simulink model "TosAeb"  (R2021a) (continued)
== system TosAeb/FcwAebJa_Step_sys/ALG_AEBSystem/ALG_AEBController/AEB_JaObjCalcApp/JAVehTgtInfoCalc/AEBJAVehObjCalc/ObjPreSelectCondCalc ==
  AEBJaVehObj : Inport  OutDataTypeStr=Bus: BusAEBJAObjBus
  subjVel : Inport  Port=2
  Bus Selector : BusSelector  OutputSignals=ObjectID,ObjectExistProb,ObjectMovingState,ObjectMovingOrientation,ObjectTypeCameraConfirmed,ObjectType,dxVariance,vx,dy,dx
  If : If  IfExpression=u1
  Merge : Merge
  ObjIsInvalidCalc : Subsystem
  TgtPreSelect : Subsystem
  TgtPreSelectCondDefault : Subsystem  TreatAsAtomicUnit=on
  ObjPreSelectCondFufilled : Outport  OutDataTypeStr=boolean  LockScale=on
  wires:
  AEBJaVehObj/1 -> Bus Selector/1
  Bus Selector/2 -> TgtPreSelect/1(ObjectExistProb)  "<ObjectExistProb>"
  Bus Selector/3 -> TgtPreSelect/2(ObjectMovingState)  "<ObjectMovingState>"
  Bus Selector/4 -> TgtPreSelect/3(ObjectMovingOrientation)  "<ObjectMovingOrientation>"
  Bus Selector/5 -> TgtPreSelect/4(ObjectTypeCameraConfirmed)  "<ObjectTypeCameraConfirmed>"
  Bus Selector/6 -> TgtPreSelect/5(ObjectType)  "<ObjectType>"
  Bus Selector/7 -> TgtPreSelect/6(dxVariance)  "<dxVariance>"
  ObjIsInvalidCalc/1(ObjIsInvalid) -> If/1
  If/1 -> TgtPreSelectCondDefault/Action
  Bus Selector/1 -> ObjIsInvalidCalc/1(ObjectID)  "<ObjectID>"
  If/2 -> TgtPreSelect/Action
  TgtPreSelect/1(ObjPreSelectCondFufilled) -> Merge/2
  Merge/1 -> ObjPreSelectCondFufilled/1
  TgtPreSelectCondDefault/1(ObjPreSelectCondFufilled) -> Merge/1
  Bus Selector/8 -> TgtPreSelect/7(vx)  "<vx>"
  Bus Selector/9 -> TgtPreSelect/8(dy)  "<dy>"
  Bus Selector/10 -> TgtPreSelect/9(dx)  "<dx>"
  subjVel/1 -> TgtPreSelect/10(subjVel)  "subjVel"

== system TosAeb/FcwAebJa_Step_sys/ALG_AEBSystem/ALG_AEBController/AEB_JaObjCalcApp/JAVehTgtInfoCalc/JAVehCollisonAssessInfoCalc/JaVehCollisonAssessInfoCalc ==
  vx : Inport  OutDataTypeStr=single
  vy : Inport  Port=2  OutDataTypeStr=single
  dx : Inport  Port=3  OutDataTypeStr=single
  dy : Inport  Port=4  OutDataTypeStr=single
  dxFrontBumper : Inport  Port=5  OutDataTypeStr=single
  tPredictTime : Inport  Port=6  OutDataTypeStr=single
  subjYawRate : Inport  Port=7  OutDataTypeStr=single
  subjVel : Inport  Port=8  OutDataTypeStr=single
  subjLongAccel : Inport  Port=9  OutDataTypeStr=single
  Action Port : ActionPort  ActionPortLabel=else
  Bus Creator : BusCreator  Inputs=3
  VehDistBrakeFullCalc : Subsystem
  VelAbsTgtCorrCalc : Subsystem
  distRadical2CollsionCalc : Subsystem
  tBrakeingCalc : Subsystem  Variant=on
  JAVehCollisonAssessInfo : Outport
  wires:
  Bus Creator/1 -> JAVehCollisonAssessInfo/1
  subjYawRate/1 -> VelAbsTgtCorrCalc/5(subjYawRate)
  subjVel/1 -> VelAbsTgtCorrCalc/6(subjVel), tBrakeingCalc/2(subjVel), VehDistBrakeFullCalc/2(subjVel)
  tPredictTime/1 -> distRadical2CollsionCalc/4(tPredictTime)
  dy/1 -> VelAbsTgtCorrCalc/3(dy)
  dxFrontBumper/1 -> VelAbsTgtCorrCalc/4(dxFrontBumper)
  vx/1 -> VelAbsTgtCorrCalc/1(vx)
  vy/1 -> VelAbsTgtCorrCalc/2(vy)
  dx/1 -> distRadical2CollsionCalc/3(dx)
  VehDistBrakeFullCalc/2(tBrake) -> tBrakeingCalc/1(tBarke)
  distRadical2CollsionCalc/2(restLatDist2Collsion) -> Bus Creator/2  "restLatDist2Collsion"
  tBrakeingCalc/1(TTB) -> Bus Creator/3  "TTB"
  VelAbsTgtCorrCalc/2(vyAbsTgtCorr) -> distRadical2CollsionCalc/2(vyAbsTgtCorr)
  VehDistBrakeFullCalc/1(distBrake) -> distRadical2CollsionCalc/5(distBrake)  "longDistBraking"
  distRadical2CollsionCalc/1(initialLatDist) -> Bus Creator/1  "initialLatDist"
  subjLongAccel/1 -> VehDistBrakeFullCalc/1(subjLongAccel)
  VelAbsTgtCorrCalc/1(vxAbsTgtCorr) -> distRadical2CollsionCalc/1(vxAbsTgtCorr)

== system TosAeb/FcwAebJa_Step_sys/ALG_AEBSystem/ALG_AEBController/AEB_JaObjCalcApp/JAVehTgtInfoCalc/JAVehCollisonAssessInfoCalc/JaVehCollisonAssessInfoDefault ==
  Action Port : ActionPort  ActionPortLabel=if(u1)
  Bus Creator : BusCreator  Inputs=3
  Constant1 : Constant  Value=255  OutDataTypeStr=single
  Constant2 : Constant  Value=255  OutDataTypeStr=single
  Constant3 : Constant  Value=255  OutDataTypeStr=single
  JAVehCollisonAssessInfo : Outport
  wires:
  Constant1/1 -> Bus Creator/1  "initialLatDist"
  Constant2/1 -> Bus Creator/2  "restLatDist2Collsion"
  Bus Creator/1 -> JAVehCollisonAssessInfo/1
  Constant3/1 -> Bus Creator/3  "TTB"

== system TosAeb/FcwAebJa_Step_sys/ALG_AEBSystem/ALG_AEBController/AEB_JaObjCalcApp/JAVehTgtInfoCalc/JAVehCollisonAssessInfoCalc/ObjIsInvalidJudge ==
  ObjectID : Inport
  Compare To Constant : simulink/Logic and Bit Operations/Compare To Constant  relop===  const=CNobjInvalidID  OutDataTypeStr=boolean
  ObjIsInvalid : Outport
  wires:
  ObjectID/1 -> Compare To Constant/1
  Compare To Constant/1 -> ObjIsInvalid/1

== system TosAeb/FcwAebJa_Step_sys/ALG_AEBSystem/ALG_AEBController/AEB_JaObjCalcApp/JAVehTgtInfoCalc/TargetFinalSelect/tgtSelect ==
  JAVehObjCalcInfo : Inport
  ObjPreSelectCondFufilled : Inport  Port=2
  subjVel : Inport  Port=3
  SteerWheelAngle_Deg : Inport  Port=4
  JAVehPrefillModSts : Inport  Port=5
  JAVehAEBfullModSts : Inport  Port=6
  Bus Selector : BusSelector  OutputSignals=AEBJAVehObj.ObjectID
  Constant : Constant  Value=P_AEB_JaVehCA_SelectSubjVelThres
  Constant1 : Constant  Value=P_AEB_JaVehCA_SelectStrWheelAngleAbsThres
  Unit Delay : UnitDelay  InitialCondition=1  SampleTime=-1  HasFrameUpgradeWarning=on
  Unit Delay1 : UnitDelay  InitialCondition=1  SampleTime=-1  HasFrameUpgradeWarning=on
  tgtObjFlow : Stateflow chart  TreatAsAtomicUnit=on
  JAVehTgtObjInfo : Outport
  wires:
  tgtObjFlow/1(JAVehTgtObjInfo) -> Bus Selector/1, JAVehTgtObjInfo/1  "tgtObj"
  JAVehObjCalcInfo/1 -> tgtObjFlow/1(JAVehObjCalcInfo)
  ObjPreSelectCondFufilled/1 -> tgtObjFlow/2(ObjPreSelectCondFufilled)
  subjVel/1 -> tgtObjFlow/3(subjVel)
  SteerWheelAngle_Deg/1 -> tgtObjFlow/4(SteerWheelAngle_Deg)
  tgtObjFlow/2(tgtIndex) -> Unit Delay/1
  Unit Delay/1 -> tgtObjFlow/10(tgtIndexK1)
  JAVehPrefillModSts/1 -> tgtObjFlow/5(JAVehAEBfullModSts)
  JAVehAEBfullModSts/1 -> tgtObjFlow/6(JAVehPrefillModSts)
  Bus Selector/1 -> Unit Delay1/1  "<ObjectID>"
  Unit Delay1/1 -> tgtObjFlow/9(TargetIDK1)
  Constant/1 -> tgtObjFlow/7(P_JaVehCA_SelectSubjVelThres)
  Constant1/1 -> tgtObjFlow/8(P_JaVehCA_SelectStrWheelAngleAbsThres)

== system TosAeb/FcwAebJa_Step_sys/ALG_AEBSystem/ALG_AEBController/AEB_JaObjCalcApp/JaCycTgtInfoCalc/JaCycCollisonAssessInfo/JaCycCollisonAssessInfoCalc ==
  vx : Inport  OutDataTypeStr=single
  vy : Inport  Port=2  OutDataTypeStr=single
  dx : Inport  Port=3  OutDataTypeStr=single
  dy : Inport  Port=4  OutDataTypeStr=single
  dxFrontBumper : Inport  Port=5  OutDataTypeStr=single
  tPredictTime : Inport  Port=6  OutDataTypeStr=single
  subjYawRate : Inport  Port=7  OutDataTypeStr=single
  subjVel : Inport  Port=8  OutDataTypeStr=single
  subjLongAccel : Inport  Port=9  OutDataTypeStr=single
  Action Port : ActionPort  ActionPortLabel=else
  Bus Creator : BusCreator  Inputs=4
  PedDistBrakeFullCalc : Subsystem
  Subsystem1 : Subsystem
  VelAbsTgtCorrCalc : Subsystem
  distRadical2CollsionCalc : Subsystem
  JaCycCollisonAssessInfo : Outport
  wires:
  Bus Creator/1 -> JaCycCollisonAssessInfo/1
  VelAbsTgtCorrCalc/2(vyAbsTgtCorr) -> distRadical2CollsionCalc/2(vyAbsTgtCorr), Subsystem1/2(vyAbsTargetCorr)
  tPredictTime/1 -> distRadical2CollsionCalc/5(tPredictTime)
  PedDistBrakeFullCalc/2(tBrake) -> Bus Creator/4  "TTB"
  subjLongAccel/1 -> PedDistBrakeFullCalc/1(subjLongAccel)
  dxFrontBumper/1 -> VelAbsTgtCorrCalc/4(dxFrontBumper)
  Subsystem1/1(TgtYawAngle) -> Bus Creator/1  "TgtYawAngle"
  subjYawRate/1 -> VelAbsTgtCorrCalc/5(subjYawRate)
  PedDistBrakeFullCalc/1(distBrake) -> Bus Creator/3  "distBraking"
  [dy] -> distRadical2CollsionCalc/4(dy)
  vy/1 -> VelAbsTgtCorrCalc/2(vy)
  dx/1 -> distRadical2CollsionCalc/3(dx)
  dy/1 -> [dy], VelAbsTgtCorrCalc/3(dy)
  distRadical2CollsionCalc/1(distRadical2Collsion) -> Bus Creator/2  "distRadical2Collsion"
  subjVel/1 -> VelAbsTgtCorrCalc/6(subjVel), PedDistBrakeFullCalc/2(subjVel)
  VelAbsTgtCorrCalc/1(vxAbsTgtCorr) -> distRadical2CollsionCalc/1(vxAbsTgtCorr), Subsystem1/1(vxAbsTargetCorr)
  vx/1 -> VelAbsTgtCorrCalc/1(vx)

== system TosAeb/FcwAebJa_Step_sys/ALG_AEBSystem/ALG_AEBController/AEB_JaObjCalcApp/JaCycTgtInfoCalc/JaCycCollisonAssessInfo/JaCycCollisonAssessInfoDefault ==
  Action Port : ActionPort  ActionPortLabel=if(u1)
  Bus Creator : BusCreator  Inputs=4
  Constant : Constant  Value=0  OutDataTypeStr=single
  Constant1 : Constant  Value=255  OutDataTypeStr=single
  Constant2 : Constant  Value=255  OutDataTypeStr=single
  Constant3 : Constant  Value=256  OutDataTypeStr=single
  JaCycCollisonAssessInfo : Outport
  wires:
  Constant2/1 -> Bus Creator/3  "distBraking"
  Constant1/1 -> Bus Creator/2  "distRadical2Collsion"
  Bus Creator/1 -> JaCycCollisonAssessInfo/1
  Constant/1 -> Bus Creator/1  "TgtYawAngle"
  Constant3/1 -> Bus Creator/4  "TTB"

== system TosAeb/FcwAebJa_Step_sys/ALG_AEBSystem/ALG_AEBController/AEB_JaObjCalcApp/JaCycTgtInfoCalc/JaCycCollisonAssessInfo/ObjIsInvalidJudge ==
  ObjectID : Inport
  Compare To Constant : simulink/Logic and Bit Operations/Compare To Constant  relop===  const=CNobjInvalidID  OutDataTypeStr=boolean
  ObjIsInvalid : Outport
  wires:
  ObjectID/1 -> Compare To Constant/1
  Compare To Constant/1 -> ObjIsInvalid/1

== system TosAeb/FcwAebJa_Step_sys/ALG_AEBSystem/ALG_AEBController/AEB_JaObjCalcApp/JaCycTgtInfoCalc/JaCycObjCalcInfoCalc/JaCycObjInfoCalc ==
  JaCycObj : Inport
  subjInfo : Inport  Port=2
  Action Port : ActionPort  ActionPortLabel=else
  Bus Creator : BusCreator  Inputs=3  OutDataTypeStr=Bus: BusAEBJaCycObjCalcInfo  NonVirtualBus=on
      settings: InheritFromInputs=off
  Bus Creator2 : BusCreator  Inputs=6  OutDataTypeStr=Bus: BusAEBJaCycObjAbsInfo  NonVirtualBus=on
      settings: InheritFromInputs=off
  Bus Selector2 : BusSelector  OutputSignals=dx,dy,vx,vy,ax,ObjectID,ObjectTypeCameraConfirmed,FR_ObjectID,FR_Dx,FR_Vy,FC_ObjectID,FC_Dx,FC_Vy
  Bus Selector3 : BusSelector  OutputSignals=subjLongAccel,subjYawRate,subjVel
  ObjCrossFlgCal : Subsystem
  SensorTgtInfo2AbsTgtInfoTrans1 : Subsystem
  "JaCycObjCalcInfo " : Outport  OutDataTypeStr=Bus: BusAEBJaCycObjCalcInfo
  wires:
  ObjCrossFlgCal/1(ObjColliInfo) -> Bus Creator/3  "BusAEBJAObjColliInfo"
  [ObjAbsInfo] -> Bus Creator/2  "BusAEBJAObjAbsInfo"
  Bus Creator2/1 -> [ObjAbsInfo], ObjCrossFlgCal/2(ObjAbsInfo)
  subjInfo/1 -> ObjCrossFlgCal/3(subjInfo), Bus Selector3/1
  JaCycObj/1 -> Bus Selector2/1, Bus Creator/1, ObjCrossFlgCal/1(JaCycObj)  "AEBJACycObj"
  Bus Creator/1 -> "JaCycObjCalcInfo "/1
  SensorTgtInfo2AbsTgtInfoTrans1/5(ObjFusQuality) -> Bus Creator2/5  "ObjFusQuality"
  Bus Selector2/8 -> SensorTgtInfo2AbsTgtInfoTrans1/8(FR_ObjectID)  "<FR_ObjectID>"
  SensorTgtInfo2AbsTgtInfoTrans1/6(ObjectYawAngleCorr) -> Bus Creator2/6  "ObjectYawAngleCorr"
  Bus Selector2/3 -> SensorTgtInfo2AbsTgtInfoTrans1/3(vx)  "<vx>"
  Bus Selector2/2 -> SensorTgtInfo2AbsTgtInfoTrans1/2(dy)  "<dy>"
  Bus Selector2/13 -> SensorTgtInfo2AbsTgtInfoTrans1/13(FC_Vy)  "<FC_Vy>"
  Bus Selector3/2 -> SensorTgtInfo2AbsTgtInfoTrans1/15(subjYawRate)  "<subjYawRate>"
  Bus Selector2/5 -> SensorTgtInfo2AbsTgtInfoTrans1/5(ax)  "<ax>"
  Bus Selector2/11 -> SensorTgtInfo2AbsTgtInfoTrans1/11(FC_ObjectID)  "<FC_ObjectID>"
  Bus Selector3/3 -> SensorTgtInfo2AbsTgtInfoTrans1/16(subjVel)  "<subjVel>"
  SensorTgtInfo2AbsTgtInfoTrans1/2(vxAbsObj) -> Bus Creator2/2  "vxAbsObj"
  Bus Selector2/6 -> SensorTgtInfo2AbsTgtInfoTrans1/6(ObjectID)  "<ObjectID>"
  Bus Selector2/1 -> SensorTgtInfo2AbsTgtInfoTrans1/1(dx)  "<dx>"
  Bus Selector3/1 -> SensorTgtInfo2AbsTgtInfoTrans1/14(subjLongAccel)  "<subjLongAccel>"
  Bus Selector2/9 -> SensorTgtInfo2AbsTgtInfoTrans1/9(FR_Dx)  "<FR_Dx>"
  SensorTgtInfo2AbsTgtInfoTrans1/4(vyRelCorr) -> Bus Creator2/4  "vyRelCorr"
  SensorTgtInfo2AbsTgtInfoTrans1/1(dxFrontBumper) -> Bus Creator2/1  "dxFrontBumper"
  Bus Selector2/7 -> SensorTgtInfo2AbsTgtInfoTrans1/7(ObjectTypeCameraConfirmed)  "<ObjectTypeCameraConfirmed>"
  SensorTgtInfo2AbsTgtInfoTrans1/3(axAbsObj) -> Bus Creator2/3  "axAbsObj"
  Bus Selector2/12 -> SensorTgtInfo2AbsTgtInfoTrans1/12(FC_Dx)  "<FC_Dx>"
  Bus Selector2/10 -> SensorTgtInfo2AbsTgtInfoTrans1/10(FR_Vy)  "<FR_Vy>"
  Bus Selector2/4 -> SensorTgtInfo2AbsTgtInfoTrans1/4(vy)  "<vy>"

== system TosAeb/FcwAebJa_Step_sys/ALG_AEBSystem/ALG_AEBController/AEB_JaObjCalcApp/JaCycTgtInfoCalc/JaCycObjCalcInfoCalc/JaCycObjInfoDefault ==
  Action Port : ActionPort  ActionPortLabel=if(u1)
  Constant : Constant  Value=JACycObjCalcInfoDefaultParams  OutDataTypeStr=Bus: BusAEBJaCycObjCalcInfo
  JaCycObjCalcInfo : Outport  OutDataTypeStr=Bus: BusAEBJaCycObjCalcInfo
  wires:
  Constant/1 -> JaCycObjCalcInfo/1

== system TosAeb/FcwAebJa_Step_sys/ALG_AEBSystem/ALG_AEBController/AEB_JaObjCalcApp/JaCycTgtInfoCalc/JaCycObjCalcInfoCalc/ObjPreSelectCondCalc ==
  JaObj : Inport  OutDataTypeStr=Bus: BusAEBJAObjBus
  Bus Selector : BusSelector  OutputSignals=ObjectID,ObjectExistProb,ObjectYawAngle,ObjectTypeCameraConfirmed,ObjectType,dxVariance,dx,dy
  If : If  IfExpression=u1
  Merge : Merge
  ObjIsInvalidCalc : Subsystem
  TgtPreSelectCondCalc : Subsystem
  TgtPreSelectCondCalc1 : Subsystem  TreatAsAtomicUnit=on
  ObjPreSelectCond : Outport  OutDataTypeStr=boolean  LockScale=on
  wires:
  JaObj/1 -> Bus Selector/1
  Bus Selector/1 -> ObjIsInvalidCalc/1(ObjectID)  "<ObjectID>"
  Bus Selector/2 -> TgtPreSelectCondCalc/1(ObjectExistProb)  "<ObjectExistProb>"
  Bus Selector/3 -> TgtPreSelectCondCalc/2(ObjectYawAngle)  "<ObjectYawAngle>"
  Bus Selector/4 -> TgtPreSelectCondCalc/3(ObjectTypeCameraComfirmed)  "<ObjectTypeCameraConfirmed>"
  Bus Selector/5 -> TgtPreSelectCondCalc/4(ObjectType)  "<ObjectType>"
  Bus Selector/6 -> TgtPreSelectCondCalc/5(dxVariance)  "<dxVariance>"
  Bus Selector/7 -> TgtPreSelectCondCalc/6(dx)  "<dx>"
  Bus Selector/8 -> TgtPreSelectCondCalc/7(dy)  "<dy>"
  TgtPreSelectCondCalc1/1(ObjPreSelectCondFufilled) -> Merge/1
  TgtPreSelectCondCalc/1(ObjPreSelectCondFufilled) -> Merge/2
  If/2 -> TgtPreSelectCondCalc/Action
  If/1 -> TgtPreSelectCondCalc1/Action
  ObjIsInvalidCalc/1(ObjIsInvalid) -> If/1
  Merge/1 -> ObjPreSelectCond/1

== system TosAeb/FcwAebJa_Step_sys/ALG_AEBSystem/ALG_AEBController/AEB_JaObjCalcApp/JaCycTgtInfoCalc/TargetSelectCalc/tgtSelectFlow ==
  JaCycObjCalcInfo : Inport
  ObjPreSelectCondFufilled : Inport  Port=2
  subjVel : Inport  Port=3
  SteerWheelAngle_Deg : Inport  Port=4
  JaCycPrefillModSts : Inport  Port=5
  JaCycAEBfullModSts : Inport  Port=6
  Bus Selector : BusSelector  OutputSignals=JaCycObj.ObjectID
  Constant : Constant  Value=P_AEB_JaCycCA_SelectStrWheelAngleAbsThres
  Constant1 : Constant  Value=P_AEB_JaCycCA_SelectSubjVelThres
  Unit Delay : UnitDelay  InitialCondition=1  SampleTime=-1  HasFrameUpgradeWarning=on
  Unit Delay1 : UnitDelay  SampleTime=-1  HasFrameUpgradeWarning=on
  tgtObjFlow : Stateflow chart  TreatAsAtomicUnit=on
  JaCycTgtObjInfo : Outport
  wires:
  JaCycObjCalcInfo/1 -> tgtObjFlow/1(JaCycObjCalcInfo)
  SteerWheelAngle_Deg/1 -> tgtObjFlow/4(SteerWheelAngle_Deg)
  Unit Delay1/1 -> tgtObjFlow/7(TargetIDK1)
  Bus Selector/1 -> Unit Delay1/1  "<ObjectID>"
  JaCycPrefillModSts/1 -> tgtObjFlow/5(JACycAEBfullModSts)
  tgtObjFlow/2(tgtIndex) -> Unit Delay/1
  Unit Delay/1 -> tgtObjFlow/8(tgtIndexK1)
  ObjPreSelectCondFufilled/1 -> tgtObjFlow/2(ObjPreSelectCondFufilled)
  subjVel/1 -> tgtObjFlow/3(subjVel)
  JaCycAEBfullModSts/1 -> tgtObjFlow/6(JACycPrefillModSts)
  tgtObjFlow/1(JaCycTgtObjInfo) -> Bus Selector/1, JaCycTgtObjInfo/1  "JaCycTgtObjInfo"
  Constant/1 -> tgtObjFlow/9(P_JaCycCA_SelectStrWheelAngleAbsThres)
  Constant1/1 -> tgtObjFlow/10(P_JaCycCA_SelectSubjVelThres)

== system TosAeb/FcwAebJa_Step_sys/ALG_AEBSystem/ALG_AEBController/AEB_LatObjInfoCalcApp/LatCycObjCalc/LatCycCollisonAssessInfoCalc/LatCycCollisonAssessInfoCalc ==
  ObjectID : Inport
  cycColliisonRiskProb : Inport  Port=2
  subLongAccel : Inport  Port=3
  subjVel : Inport  Port=4
  Action Port : ActionPort  ActionPortLabel=else
  Bus Creator : BusCreator  Inputs=4
  DistBrkCondCalc : Subsystem
  LatCollsionSeverityJudge : Subsystem
  latCollisonAssessInfo : Outport
  wires:
  ObjectID/1 -> LatCollsionSeverityJudge/1(ObjectID)
  cycColliisonRiskProb/1 -> LatCollsionSeverityJudge/2(cycColliisonRiskProb)
  subLongAccel/1 -> DistBrkCondCalc/1(subLongAccel)
  subjVel/1 -> DistBrkCondCalc/2(subjVel)
  LatCollsionSeverityJudge/1(latCollsionSeverity) -> Bus Creator/1  "latCollisionSeverity"
  Bus Creator/1 -> latCollisonAssessInfo/1  "latCollisonAssessInfo"
  DistBrkCondCalc/2(distBraking4FullBrake) -> Bus Creator/3  "distBraking4FullBrake"
  DistBrkCondCalc/3(distSecure) -> Bus Creator/4  "distSecure"
  DistBrkCondCalc/1(distBraking4PartBrake) -> Bus Creator/2  "distBraking4PartBrake"

== system TosAeb/FcwAebJa_Step_sys/ALG_AEBSystem/ALG_AEBController/AEB_LatObjInfoCalcApp/LatCycObjCalc/LatCycCollisonAssessInfoCalc/LatCycCollisonAssessInfoDefault ==
  Action Port : ActionPort  ActionPortLabel=if(u1)
  Bus Creator : BusCreator  Inputs=4
  Constant : Constant  Value=CollisionSeverity.None
  Constant1 : Constant  Value=CNNull
  Constant2 : Constant  Value=CNNull
  Constant3 : Constant  Value=CNNull
  latCollisonAssessInfo : Outport
  wires:
  Constant/1 -> Bus Creator/1  "latCollisionSeverity"
  Bus Creator/1 -> latCollisonAssessInfo/1  "latCollisonAssessInfo"
  Constant2/1 -> Bus Creator/4  "distSecure"
  Constant1/1 -> Bus Creator/2  "distBraking4PartBrake"
  Constant3/1 -> Bus Creator/3  "distBraking4FullBrake"

== system TosAeb/FcwAebJa_Step_sys/ALG_AEBSystem/ALG_AEBController/AEB_LatObjInfoCalcApp/LatCycObjCalc/LatCycCollisonAssessInfoCalc/ObjIsInvalidJudge ==
  ObjectID : Inport
  Compare To Constant : simulink/Logic and Bit Operations/Compare To Constant  relop===  const=CNobjInvalidID  OutDataTypeStr=boolean
  ObjIsInvalid : Outport
  wires:
  ObjectID/1 -> Compare To Constant/1
  Compare To Constant/1 -> ObjIsInvalid/1

== system TosAeb/FcwAebJa_Step_sys/ALG_AEBSystem/ALG_AEBController/AEB_LatObjInfoCalcApp/LatCycObjCalc/LatCycObjCalc/TgtPreSelectCalc ==
  latCycObjInfo : Inport  OutDataTypeStr=Bus: BusAEBLatObj  PortDimensions=1  BusOutputAsStruct=on
  subjVel : Inport  Port=2
  Bus Selector : BusSelector  OutputSignals=ObjectID,dx,dy,ObjectExistProb,dyVariance,ObjectMovingOrientation,ObjectType,ObjectTypeCameraConfirmed,vx
  If : If  IfExpression=u1
  Merge : Merge
  ObjIsInvalidJudge : Subsystem
  TgtPreSelect : Subsystem
  TgtPreSelectDefault : Subsystem  TreatAsAtomicUnit=on
  ObjPreSelectCond : Outport
  wires:
  If/2 -> TgtPreSelect/Action
  Merge/1 -> ObjPreSelectCond/1
  TgtPreSelect/1(ObjPreSelectCond) -> Merge/2
  TgtPreSelectDefault/1(ObjPreSelectCond) -> Merge/1
  If/1 -> TgtPreSelectDefault/Action
  Bus Selector/1 -> ObjIsInvalidJudge/1(ObjectID)  "<ObjectID>"
  ObjIsInvalidJudge/1(ObjIsInvalid) -> If/1
  latCycObjInfo/1 -> Bus Selector/1
  Bus Selector/2 -> TgtPreSelect/1(dx)  "<dx>"
  Bus Selector/3 -> TgtPreSelect/2(dy)  "<dy>"
  Bus Selector/4 -> TgtPreSelect/3(ObjectExistProb)  "<ObjectExistProb>"
  Bus Selector/5 -> TgtPreSelect/4(dyVariance)  "<dyVariance>"
  Bus Selector/6 -> TgtPreSelect/5(ObjectMovingOrientation)  "<ObjectMovingOrientation>"
  Bus Selector/7 -> TgtPreSelect/6(ObjectType)  "<ObjectType>"
  Bus Selector/8 -> TgtPreSelect/7(ObjectTypeCameraConfirmed)  "<ObjectTypeCameraConfirmed>"
  Bus Selector/9 -> TgtPreSelect/8(vx)  "<vx>"
  subjVel/1 -> TgtPreSelect/9(subjVel)

== system TosAeb/FcwAebJa_Step_sys/ALG_AEBSystem/ALG_AEBController/AEB_LatObjInfoCalcApp/LatCycObjCalc/LatCycObjCalc/latCycObjInfoCalc ==
  subjInfo : Inport
  latCycObjInfo : Inport  Port=2  OutDataTypeStr=Bus: BusAEBLatObj
  Action Port : ActionPort  ActionPortLabel=else
  Bus Creator : BusCreator  Inputs=6  OutDataTypeStr=Bus: BusLatCycObjCalcInfo  NonVirtualBus=on
      settings: InheritFromInputs=off
  Bus Creator1 : BusCreator  Inputs=5
  Bus Selector : BusSelector  OutputSignals=dx,vx,ax,vy,ObjectID,ObjectTypeCameraConfirmed,dy,FR_ObjectID,FR_Dx,FR_Dy,FR_Vy,FC_ObjectID,FC_Dx,FC_Dy,FC_Vy
  Bus Selector1 : BusSelector  OutputSignals=subjVel,subjLongAccel,subjYawRate
  Constant : Constant  Value=TRUE
  Constant1 : Constant  Value=P_AEB_LatCycCA_PreCollisionConfig
  ObjColiisionRiskProbCalc : Subsystem
  ObjCrossFlgCal : Subsystem
  SensorTgtInfo2AbsTgtInfoTrans : Subsystem
  Switch : Switch  Criteria=u2 ~= 0
  Terminator : Terminator
  Terminator1 : Terminator
  latCycCalcInfo : Outport  OutDataTypeStr=Bus: BusLatCycObjCalcInfo
  wires:
  [ObjAbsInfo] -> ObjCrossFlgCal/3(ObjAbsInfo)
  ObjColiisionRiskProbCalc/3(dyCollisionRiskProbOut) -> Terminator1/1
  ObjColiisionRiskProbCalc/2(dxCollisionRiskProbOut) -> Terminator/1
  subjInfo/1 -> ObjColiisionRiskProbCalc/3(subjInfo), ObjCrossFlgCal/1(subjInfo), Bus Selector1/1
  Bus Creator1/1 -> ObjColiisionRiskProbCalc/2(ObjAbsInfo), [ObjAbsInfo]
  Bus Creator/1 -> latCycCalcInfo/1
  ObjColiisionRiskProbCalc/1(cycCollisionRiskProb) -> Bus Creator/3  "cycCollisionRiskProb"
  [ObjAbsInfo] -> Bus Creator/2  "cycObjAbsInfo"
  Switch/1 -> Bus Creator/5  "cycPreCollisionFlg"
  latCycObjInfo/1 -> ObjCrossFlgCal/2(ObjInfoRaw), Bus Selector/1, ObjColiisionRiskProbCalc/1(ObjInfoRaw), Bus Creator/1  "latCycObjInfo"
  Bus Selector/11 -> SensorTgtInfo2AbsTgtInfoTrans/11(FR_Vy)  "<FR_Vy>"
  Bus Selector/6 -> SensorTgtInfo2AbsTgtInfoTrans/6(ObjectTypeCameraConfirmed)  "<ObjectTypeCameraConfirmed>"
  Bus Selector/8 -> SensorTgtInfo2AbsTgtInfoTrans/8(FR_ObjectID)  "<FR_ObjectID>"
  Bus Selector/5 -> SensorTgtInfo2AbsTgtInfoTrans/5(ObjectID)  "<ObjectID>"
  SensorTgtInfo2AbsTgtInfoTrans/4(vyCorr) -> Bus Creator1/4  "vyCorr"
  Bus Selector1/3 -> SensorTgtInfo2AbsTgtInfoTrans/18(subjYawRate)  "<subjYawRate>"
  Bus Selector1/1 -> SensorTgtInfo2AbsTgtInfoTrans/16(subjVel)  "<subjVel>"
  SensorTgtInfo2AbsTgtInfoTrans/5(ObjFusQuality) -> Bus Creator1/5  "ObjFusQuality"
  SensorTgtInfo2AbsTgtInfoTrans/2(vxAbsObj) -> Bus Creator1/2  "vxAbsObj"
  SensorTgtInfo2AbsTgtInfoTrans/1(dxFrontBumper) -> Bus Creator1/1  "dxFrontBumper"
  SensorTgtInfo2AbsTgtInfoTrans/3(axAbsObj) -> Bus Creator1/3  "axAbsObj"
  Bus Selector/3 -> SensorTgtInfo2AbsTgtInfoTrans/3(ax)  "<ax>"
  Bus Selector/1 -> SensorTgtInfo2AbsTgtInfoTrans/1(dx)  "<dx>"
  Bus Selector/4 -> SensorTgtInfo2AbsTgtInfoTrans/4(vy)  "<vy>"
  Bus Selector/2 -> SensorTgtInfo2AbsTgtInfoTrans/2(vx)  "<vx>"
  Bus Selector1/2 -> SensorTgtInfo2AbsTgtInfoTrans/17(subjLongAccel)  "<subjLongAccel>"
  Bus Selector/12 -> SensorTgtInfo2AbsTgtInfoTrans/12(FC_ObjectID)  "<FC_ObjectID>"
  Bus Selector/15 -> SensorTgtInfo2AbsTgtInfoTrans/15(FC_Vy)  "<FC_Vy>"
  Bus Selector/9 -> SensorTgtInfo2AbsTgtInfoTrans/9(FR_Dx)  "<FR_Dx>"
  Bus Selector/10 -> SensorTgtInfo2AbsTgtInfoTrans/10(FR_Dy)  "<FR_Dy>"
  Bus Selector/13 -> SensorTgtInfo2AbsTgtInfoTrans/13(FC_Dx)  "<FC_Dx>"
  Bus Selector/14 -> SensorTgtInfo2AbsTgtInfoTrans/14(FC_Dy)  "<FC_Dy>"
  Bus Selector/7 -> SensorTgtInfo2AbsTgtInfoTrans/7(dy)  "<dy>"
  Constant/1 -> Switch/3
  ObjCrossFlgCal/1(cycPreCollisionFlg) -> Switch/1  "cycPreCollisionFlg"
  Constant1/1 -> Switch/2
  ObjColiisionRiskProbCalc/4(preCollisonMaxTime) -> Bus Creator/4  "cycCollisionRiskProb_TTC"
  ObjCrossFlgCal/2(TTC) -> Bus Creator/6  "cycPreCollisionFlg_TTC"

== system TosAeb/FcwAebJa_Step_sys/ALG_AEBSystem/ALG_AEBController/AEB_LatObjInfoCalcApp/LatCycObjCalc/LatCycObjCalc/latCycObjInfoDefault ==
  Action Port : ActionPort  ActionPortLabel=if(u1)
  LatObjCalcInfoDefaultParams : Constant  Value=latCycObjCalcInfoDefaultParams  OutDataTypeStr=Bus: BusLatCycObjCalcInfo
  latCycCalcInfo : Outport  OutDataTypeStr=Bus: BusLatCycObjCalcInfo
  wires:
  LatObjCalcInfoDefaultParams/1 -> latCycCalcInfo/1

== system TosAeb/FcwAebJa_Step_sys/ALG_AEBSystem/ALG_AEBController/AEB_LatObjInfoCalcApp/LatPedObjCalc/LatPedCollisonAssessInfo/LatPedCollisonAssessInfo ==
  ObjectID : Inport
  pedColliisonRisk : Inport  Port=2  OutDataTypeStr=single
  dxFrontBumper : Inport  Port=3  OutDataTypeStr=single
  vx : Inport  Port=4
  subjVel : Inport  Port=5
  subLongAccel : Inport  Port=6  OutDataTypeStr=single
  FcwHMISetting : Inport  Port=7  OutDataTypeStr=Enum: FCWSnvty
  Action Port : ActionPort  ActionPortLabel=else
  Bus Creator : BusCreator  Inputs=3  OutDataTypeStr=Bus: BusLatPedCollisionAssessInfo
      settings: InheritFromInputs=off
  LatPedCollisionSeverityCalc : Subsystem
  LatPedLongDistCondCalc : Subsystem
  latPedCollisonAssessInfo : Outport
  wires:
  ObjectID/1 -> LatPedCollisionSeverityCalc/1(ObjectID)
  subjVel/1 -> LatPedLongDistCondCalc/1(subjVel), LatPedCollisionSeverityCalc/5(subjVel)
  subLongAccel/1 -> LatPedLongDistCondCalc/2(subLongAccel)
  pedColliisonRisk/1 -> LatPedCollisionSeverityCalc/2(pedColliisonRisk)
  LatPedLongDistCondCalc/1(distBraking) -> [distBraking], LatPedCollisionSeverityCalc/7(distBraking)  "distBraking"
  dxFrontBumper/1 -> LatPedCollisionSeverityCalc/3(dxFrontBumper)
  FcwHMISetting/1 -> LatPedCollisionSeverityCalc/6(FcwHMISetting)
  Bus Creator/1 -> latPedCollisonAssessInfo/1
  [distBraking] -> Bus Creator/2  "distBraking"
  LatPedCollisionSeverityCalc/1(latCollsionSeverity) -> Bus Creator/1  "latCollsionSeverity"
  LatPedLongDistCondCalc/2(distSecure) -> Bus Creator/3, LatPedCollisionSeverityCalc/8(distSecure)  "distSecure"
  vx/1 -> LatPedCollisionSeverityCalc/4(vx)

== system TosAeb/FcwAebJa_Step_sys/ALG_AEBSystem/ALG_AEBController/AEB_LatObjInfoCalcApp/LatPedObjCalc/LatPedCollisonAssessInfo/LatPedCollisonAssessInfoDefault ==
  Action Port : ActionPort  ActionPortLabel=if(u1)
  Bus Creator : BusCreator  Inputs=3  OutDataTypeStr=Bus: BusLatPedCollisionAssessInfo
      settings: InheritFromInputs=off
  Constant : Constant  Value=CollisionSeverity.None
  Constant1 : Constant  Value=CNNull
  Constant2 : Constant  Value=CNNull
  latPedCollisonAssessInfo : Outport
  wires:
  Constant2/1 -> Bus Creator/3  "distSecure"
  Constant1/1 -> Bus Creator/2  "distBraking"
  Bus Creator/1 -> latPedCollisonAssessInfo/1
  Constant/1 -> Bus Creator/1  "latCollsionSeverity"
